simulink model "test03"  (R2022a)
settings: Solver=FixedStepAuto  StartTime=0.0  StopTime=0.05  FixedStep=1e-4  RelTol=1e-3
values from neighbouring files (symbols this model uses):
  A = 0.01    [parameter_load3.m]
  d = 2.6    [parameter_load3.m]
  I = 3055e-8    (= 3.055e-05)    [parameter_load3.m]
  jv = 1064400    [parameter_load3.m]
  kf = 6.67e7    (= 66700000)    [parameter_load3.m]
  kv = 400000    [parameter_load3.m]
  L = 210    [parameter_load3.m]
  m = 7800*(7684e-6)    (= 59.9352)    [parameter_load3.m]
  mv = 42400    [parameter_load3.m]
  position = 0.5*L    (= 105)    [parameter_load3.m]
  r = 840/1000/2    (= 0.42)    [parameter_load3.m]
  v = 100/3.6    (= 27.7777777778)    [parameter_load3.m]
(part 2 of 73)

== system test03/Subsystem3/Subsystem1/Subsystem17 ==
  index : Inport
  F : Inport  Port=2
  Add1 : Sum
  Constant2 : Constant  Value=2
  Constant6 : Constant
  Constant7 : Constant  Value=2
  Constant8 : Constant  Value=2
  Demux : Demux  Outputs=2
  Subsystem : Subsystem
  Subsystem1 : Subsystem
  Subsystem2 : Subsystem
  Subsystem3 : Subsystem
  Out1 : Outport
  wires:
  Subsystem3/1(Out1) -> Add1/2
  Subsystem2/1(Out1) -> Add1/1
  Subsystem1/8(Omiga_n) -> Subsystem3/8(Omiga_n)
  Subsystem1/7(Omiga_bn) -> Subsystem3/7(Omiga_bn)
  Subsystem1/6(static_n) -> Subsystem3/6(static_n)
  Subsystem1/5(Sn) -> Subsystem3/5(Sn)
  Subsystem1/4(theta_n3) -> Subsystem3/4(theta_n3)
  Subsystem1/3(theta_n2) -> Subsystem3/3(theta_n2)
  Subsystem1/2(theta_n1) -> Subsystem3/2(theta_n1)
  Subsystem1/1(An1) -> Subsystem3/1(An1)
  Subsystem/8(Omiga_n) -> Subsystem2/8(Omiga_n)
  Subsystem/7(Omiga_bn) -> Subsystem2/7(Omiga_bn)
  Subsystem/6(static_n) -> Subsystem2/6(static_n)
  Subsystem/5(Sn) -> Subsystem2/5(Sn)
  Subsystem/1(An1) -> Subsystem2/1(An1)
  Subsystem/4(theta_n3) -> Subsystem2/4(theta_n3)
  Subsystem/3(theta_n2) -> Subsystem2/3(theta_n2)
  Subsystem/2(theta_n1) -> Subsystem2/2(theta_n1)
  Demux/2 -> Subsystem1/4(F)
  Demux/1 -> Subsystem/4(F)
  F/1 -> Demux/1
  Constant7/1 -> Subsystem1/2(r)
  Constant8/1 -> Subsystem1/3(k)
  Constant6/1 -> Subsystem/3(k)
  Constant2/1 -> Subsystem/2(r)
  Add1/1 -> Out1/1
  index/1 -> Subsystem1/1(imod), Subsystem/1(imod)

== system test03/Subsystem3/Subsystem1/Subsystem18 ==
  index : Inport
  F : Inport  Port=2
  Add1 : Sum
  Constant2 : Constant  Value=2
  Constant6 : Constant
  Constant7 : Constant  Value=2
  Constant8 : Constant  Value=2
  Demux : Demux  Outputs=2
  Subsystem : Subsystem
  Subsystem1 : Subsystem
  Subsystem2 : Subsystem
  Subsystem3 : Subsystem
  Out1 : Outport
  wires:
  Subsystem3/1(Out1) -> Add1/2
  Subsystem2/1(Out1) -> Add1/1
  Subsystem1/8(Omiga_n) -> Subsystem3/8(Omiga_n)
  Subsystem1/7(Omiga_bn) -> Subsystem3/7(Omiga_bn)
  Subsystem1/6(static_n) -> Subsystem3/6(static_n)
  Subsystem1/5(Sn) -> Subsystem3/5(Sn)
  Subsystem1/4(theta_n3) -> Subsystem3/4(theta_n3)
  Subsystem1/3(theta_n2) -> Subsystem3/3(theta_n2)
  Subsystem1/2(theta_n1) -> Subsystem3/2(theta_n1)
  Subsystem1/1(An1) -> Subsystem3/1(An1)
  Subsystem/8(Omiga_n) -> Subsystem2/8(Omiga_n)
  Subsystem/7(Omiga_bn) -> Subsystem2/7(Omiga_bn)
  Subsystem/6(static_n) -> Subsystem2/6(static_n)
  Subsystem/5(Sn) -> Subsystem2/5(Sn)
  Subsystem/1(An1) -> Subsystem2/1(An1)
  Subsystem/4(theta_n3) -> Subsystem2/4(theta_n3)
  Subsystem/3(theta_n2) -> Subsystem2/3(theta_n2)
  Subsystem/2(theta_n1) -> Subsystem2/2(theta_n1)
  Demux/2 -> Subsystem1/4(F)
  Demux/1 -> Subsystem/4(F)
  F/1 -> Demux/1
  Constant7/1 -> Subsystem1/2(r)
  Constant8/1 -> Subsystem1/3(k)
  Constant6/1 -> Subsystem/3(k)
  Constant2/1 -> Subsystem/2(r)
  Add1/1 -> Out1/1
  index/1 -> Subsystem1/1(imod), Subsystem/1(imod)

== system test03/Subsystem3/Subsystem1/Subsystem19 ==
  index : Inport
  F : Inport  Port=2
  Add1 : Sum
  Constant2 : Constant  Value=2
  Constant6 : Constant
  Constant7 : Constant  Value=2
  Constant8 : Constant  Value=2
  Demux : Demux  Outputs=2
  Subsystem : Subsystem
  Subsystem1 : Subsystem
  Subsystem2 : Subsystem
  Subsystem3 : Subsystem
  Out1 : Outport
  wires:
  index/1 -> Subsystem/1(imod), Subsystem1/1(imod)
  Add1/1 -> Out1/1
  Constant2/1 -> Subsystem/2(r)
  Constant6/1 -> Subsystem/3(k)
  Constant8/1 -> Subsystem1/3(k)
  Constant7/1 -> Subsystem1/2(r)
  F/1 -> Demux/1
  Demux/1 -> Subsystem/4(F)
  Demux/2 -> Subsystem1/4(F)
  Subsystem/2(theta_n1) -> Subsystem2/2(theta_n1)
  Subsystem/3(theta_n2) -> Subsystem2/3(theta_n2)
  Subsystem/4(theta_n3) -> Subsystem2/4(theta_n3)
  Subsystem/1(An1) -> Subsystem2/1(An1)
  Subsystem/5(Sn) -> Subsystem2/5(Sn)
  Subsystem/6(static_n) -> Subsystem2/6(static_n)
  Subsystem/7(Omiga_bn) -> Subsystem2/7(Omiga_bn)
  Subsystem/8(Omiga_n) -> Subsystem2/8(Omiga_n)
  Subsystem1/1(An1) -> Subsystem3/1(An1)
  Subsystem1/2(theta_n1) -> Subsystem3/2(theta_n1)
  Subsystem1/3(theta_n2) -> Subsystem3/3(theta_n2)
  Subsystem1/4(theta_n3) -> Subsystem3/4(theta_n3)
  Subsystem1/5(Sn) -> Subsystem3/5(Sn)
  Subsystem1/6(static_n) -> Subsystem3/6(static_n)
  Subsystem1/7(Omiga_bn) -> Subsystem3/7(Omiga_bn)
  Subsystem1/8(Omiga_n) -> Subsystem3/8(Omiga_n)
  Subsystem2/1(Out1) -> Add1/1
  Subsystem3/1(Out1) -> Add1/2

== system test03/Subsystem3/Subsystem1/Subsystem2 ==
  index : Inport
  F : Inport  Port=2
  Add1 : Sum
  Constant2 : Constant  Value=2
  Constant6 : Constant
  Constant7 : Constant  Value=2
  Constant8 : Constant  Value=2
  Demux : Demux  Outputs=2
  Subsystem : Subsystem
  Subsystem1 : Subsystem
  Subsystem2 : Subsystem
  Subsystem3 : Subsystem
  Out1 : Outport
  wires:
  index/1 -> Subsystem/1(imod), Subsystem1/1(imod)
  Add1/1 -> Out1/1
  Constant2/1 -> Subsystem/2(r)
  Constant6/1 -> Subsystem/3(k)
  Constant8/1 -> Subsystem1/3(k)
  Constant7/1 -> Subsystem1/2(r)
  F/1 -> Demux/1
  Demux/1 -> Subsystem/4(F)
  Demux/2 -> Subsystem1/4(F)
  Subsystem/2(theta_n1) -> Subsystem2/2(theta_n1)
  Subsystem/3(theta_n2) -> Subsystem2/3(theta_n2)
  Subsystem/4(theta_n3) -> Subsystem2/4(theta_n3)
  Subsystem/1(An1) -> Subsystem2/1(An1)
  Subsystem/5(Sn) -> Subsystem2/5(Sn)
  Subsystem/6(static_n) -> Subsystem2/6(static_n)
  Subsystem/7(Omiga_bn) -> Subsystem2/7(Omiga_bn)
  Subsystem/8(Omiga_n) -> Subsystem2/8(Omiga_n)
  Subsystem1/1(An1) -> Subsystem3/1(An1)
  Subsystem1/2(theta_n1) -> Subsystem3/2(theta_n1)
  Subsystem1/3(theta_n2) -> Subsystem3/3(theta_n2)
  Subsystem1/4(theta_n3) -> Subsystem3/4(theta_n3)
  Subsystem1/5(Sn) -> Subsystem3/5(Sn)
  Subsystem1/6(static_n) -> Subsystem3/6(static_n)
  Subsystem1/7(Omiga_bn) -> Subsystem3/7(Omiga_bn)
  Subsystem1/8(Omiga_n) -> Subsystem3/8(Omiga_n)
  Subsystem2/1(Out1) -> Add1/1
  Subsystem3/1(Out1) -> Add1/2

== system test03/Subsystem3/Subsystem1/Subsystem20 ==
  index : Inport
  F : Inport  Port=2
  Add1 : Sum
  Constant2 : Constant  Value=2
  Constant6 : Constant
  Constant7 : Constant  Value=2
  Constant8 : Constant  Value=2
  Demux : Demux  Outputs=2
  Subsystem : Subsystem
  Subsystem1 : Subsystem
  Subsystem2 : Subsystem
  Subsystem3 : Subsystem
  Out1 : Outport
  wires:
  Subsystem3/1(Out1) -> Add1/2
  Subsystem2/1(Out1) -> Add1/1
  Subsystem1/8(Omiga_n) -> Subsystem3/8(Omiga_n)
  Subsystem1/7(Omiga_bn) -> Subsystem3/7(Omiga_bn)
  Subsystem1/6(static_n) -> Subsystem3/6(static_n)
  Subsystem1/5(Sn) -> Subsystem3/5(Sn)
  Subsystem1/4(theta_n3) -> Subsystem3/4(theta_n3)
  Subsystem1/3(theta_n2) -> Subsystem3/3(theta_n2)
  Subsystem1/2(theta_n1) -> Subsystem3/2(theta_n1)
  Subsystem1/1(An1) -> Subsystem3/1(An1)
  Subsystem/8(Omiga_n) -> Subsystem2/8(Omiga_n)
  Subsystem/7(Omiga_bn) -> Subsystem2/7(Omiga_bn)
  Subsystem/6(static_n) -> Subsystem2/6(static_n)
  Subsystem/5(Sn) -> Subsystem2/5(Sn)
  Subsystem/1(An1) -> Subsystem2/1(An1)
  Subsystem/4(theta_n3) -> Subsystem2/4(theta_n3)
  Subsystem/3(theta_n2) -> Subsystem2/3(theta_n2)
  Subsystem/2(theta_n1) -> Subsystem2/2(theta_n1)
  Demux/2 -> Subsystem1/4(F)
  Demux/1 -> Subsystem/4(F)
  F/1 -> Demux/1
  Constant7/1 -> Subsystem1/2(r)
  Constant8/1 -> Subsystem1/3(k)
  Constant6/1 -> Subsystem/3(k)
  Constant2/1 -> Subsystem/2(r)
  Add1/1 -> Out1/1
  index/1 -> Subsystem1/1(imod), Subsystem/1(imod)

== system test03/Subsystem3/Subsystem1/Subsystem3 ==
  index : Inport
  F : Inport  Port=2
  Add1 : Sum
  Constant2 : Constant  Value=2
  Constant6 : Constant
  Constant7 : Constant  Value=2
  Constant8 : Constant  Value=2
  Demux : Demux  Outputs=2
  Subsystem : Subsystem
  Subsystem1 : Subsystem
  Subsystem2 : Subsystem
  Subsystem3 : Subsystem
  Out1 : Outport
  wires:
  Subsystem3/1(Out1) -> Add1/2
  Subsystem2/1(Out1) -> Add1/1
  Subsystem1/8(Omiga_n) -> Subsystem3/8(Omiga_n)
  Subsystem1/7(Omiga_bn) -> Subsystem3/7(Omiga_bn)
  Subsystem1/6(static_n) -> Subsystem3/6(static_n)
  Subsystem1/5(Sn) -> Subsystem3/5(Sn)
  Subsystem1/4(theta_n3) -> Subsystem3/4(theta_n3)
  Subsystem1/3(theta_n2) -> Subsystem3/3(theta_n2)
  Subsystem1/2(theta_n1) -> Subsystem3/2(theta_n1)
  Subsystem1/1(An1) -> Subsystem3/1(An1)
  Subsystem/8(Omiga_n) -> Subsystem2/8(Omiga_n)
  Subsystem/7(Omiga_bn) -> Subsystem2/7(Omiga_bn)
  Subsystem/6(static_n) -> Subsystem2/6(static_n)
  Subsystem/5(Sn) -> Subsystem2/5(Sn)
  Subsystem/1(An1) -> Subsystem2/1(An1)
  Subsystem/4(theta_n3) -> Subsystem2/4(theta_n3)
  Subsystem/3(theta_n2) -> Subsystem2/3(theta_n2)
  Subsystem/2(theta_n1) -> Subsystem2/2(theta_n1)
  Demux/2 -> Subsystem1/4(F)
  Demux/1 -> Subsystem/4(F)
  F/1 -> Demux/1
  Constant7/1 -> Subsystem1/2(r)
  Constant8/1 -> Subsystem1/3(k)
  Constant6/1 -> Subsystem/3(k)
  Constant2/1 -> Subsystem/2(r)
  Add1/1 -> Out1/1
  index/1 -> Subsystem1/1(imod), Subsystem/1(imod)

== system test03/Subsystem3/Subsystem1/Subsystem4 ==
  index : Inport
  F : Inport  Port=2
  Add1 : Sum
  Constant2 : Constant  Value=2
  Constant6 : Constant
  Constant7 : Constant  Value=2
  Constant8 : Constant  Value=2
  Demux : Demux  Outputs=2
  Subsystem : Subsystem
  Subsystem1 : Subsystem
  Subsystem2 : Subsystem
  Subsystem3 : Subsystem
  Out1 : Outport
  wires:
  index/1 -> Subsystem/1(imod), Subsystem1/1(imod)
  Add1/1 -> Out1/1
  Constant2/1 -> Subsystem/2(r)
  Constant6/1 -> Subsystem/3(k)
  Constant8/1 -> Subsystem1/3(k)
  Constant7/1 -> Subsystem1/2(r)
  F/1 -> Demux/1
  Demux/1 -> Subsystem/4(F)
  Demux/2 -> Subsystem1/4(F)
  Subsystem/2(theta_n1) -> Subsystem2/2(theta_n1)
  Subsystem/3(theta_n2) -> Subsystem2/3(theta_n2)
  Subsystem/4(theta_n3) -> Subsystem2/4(theta_n3)
  Subsystem/1(An1) -> Subsystem2/1(An1)
  Subsystem/5(Sn) -> Subsystem2/5(Sn)
  Subsystem/6(static_n) -> Subsystem2/6(static_n)
  Subsystem/7(Omiga_bn) -> Subsystem2/7(Omiga_bn)
  Subsystem/8(Omiga_n) -> Subsystem2/8(Omiga_n)
  Subsystem1/1(An1) -> Subsystem3/1(An1)
  Subsystem1/2(theta_n1) -> Subsystem3/2(theta_n1)
  Subsystem1/3(theta_n2) -> Subsystem3/3(theta_n2)
  Subsystem1/4(theta_n3) -> Subsystem3/4(theta_n3)
  Subsystem1/5(Sn) -> Subsystem3/5(Sn)
  Subsystem1/6(static_n) -> Subsystem3/6(static_n)
  Subsystem1/7(Omiga_bn) -> Subsystem3/7(Omiga_bn)
  Subsystem1/8(Omiga_n) -> Subsystem3/8(Omiga_n)
  Subsystem2/1(Out1) -> Add1/1
  Subsystem3/1(Out1) -> Add1/2

== system test03/Subsystem3/Subsystem1/Subsystem5 ==
  index : Inport
  F : Inport  Port=2
  Add1 : Sum
  Constant2 : Constant  Value=2
  Constant6 : Constant
  Constant7 : Constant  Value=2
  Constant8 : Constant  Value=2
  Demux : Demux  Outputs=2
  Subsystem : Subsystem
  Subsystem1 : Subsystem
  Subsystem2 : Subsystem
  Subsystem3 : Subsystem
  Out1 : Outport
  wires:
  Subsystem3/1(Out1) -> Add1/2
  Subsystem2/1(Out1) -> Add1/1
  Subsystem1/8(Omiga_n) -> Subsystem3/8(Omiga_n)
  Subsystem1/7(Omiga_bn) -> Subsystem3/7(Omiga_bn)
  Subsystem1/6(static_n) -> Subsystem3/6(static_n)
  Subsystem1/5(Sn) -> Subsystem3/5(Sn)
  Subsystem1/4(theta_n3) -> Subsystem3/4(theta_n3)
  Subsystem1/3(theta_n2) -> Subsystem3/3(theta_n2)
  Subsystem1/2(theta_n1) -> Subsystem3/2(theta_n1)
  Subsystem1/1(An1) -> Subsystem3/1(An1)
  Subsystem/8(Omiga_n) -> Subsystem2/8(Omiga_n)
  Subsystem/7(Omiga_bn) -> Subsystem2/7(Omiga_bn)
  Subsystem/6(static_n) -> Subsystem2/6(static_n)
  Subsystem/5(Sn) -> Subsystem2/5(Sn)
  Subsystem/1(An1) -> Subsystem2/1(An1)
  Subsystem/4(theta_n3) -> Subsystem2/4(theta_n3)
  Subsystem/3(theta_n2) -> Subsystem2/3(theta_n2)
  Subsystem/2(theta_n1) -> Subsystem2/2(theta_n1)
  Demux/2 -> Subsystem1/4(F)
  Demux/1 -> Subsystem/4(F)
  F/1 -> Demux/1
  Constant7/1 -> Subsystem1/2(r)
  Constant8/1 -> Subsystem1/3(k)
  Constant6/1 -> Subsystem/3(k)
  Constant2/1 -> Subsystem/2(r)
  Add1/1 -> Out1/1
  index/1 -> Subsystem1/1(imod), Subsystem/1(imod)

== system test03/Subsystem3/Subsystem1/Subsystem6 ==
  index : Inport
  F : Inport  Port=2
  Add1 : Sum
  Constant2 : Constant  Value=2
  Constant6 : Constant
  Constant7 : Constant  Value=2
  Constant8 : Constant  Value=2
  Demux : Demux  Outputs=2
  Subsystem : Subsystem
  Subsystem1 : Subsystem
  Subsystem2 : Subsystem
  Subsystem3 : Subsystem
  Out1 : Outport
  wires:
  index/1 -> Subsystem/1(imod), Subsystem1/1(imod)
  Add1/1 -> Out1/1
  Constant2/1 -> Subsystem/2(r)
  Constant6/1 -> Subsystem/3(k)
  Constant8/1 -> Subsystem1/3(k)
  Constant7/1 -> Subsystem1/2(r)
  F/1 -> Demux/1
  Demux/1 -> Subsystem/4(F)
  Demux/2 -> Subsystem1/4(F)
  Subsystem/2(theta_n1) -> Subsystem2/2(theta_n1)
  Subsystem/3(theta_n2) -> Subsystem2/3(theta_n2)
  Subsystem/4(theta_n3) -> Subsystem2/4(theta_n3)
  Subsystem/1(An1) -> Subsystem2/1(An1)
  Subsystem/5(Sn) -> Subsystem2/5(Sn)
  Subsystem/6(static_n) -> Subsystem2/6(static_n)
  Subsystem/7(Omiga_bn) -> Subsystem2/7(Omiga_bn)
  Subsystem/8(Omiga_n) -> Subsystem2/8(Omiga_n)
  Subsystem1/1(An1) -> Subsystem3/1(An1)
  Subsystem1/2(theta_n1) -> Subsystem3/2(theta_n1)
  Subsystem1/3(theta_n2) -> Subsystem3/3(theta_n2)
  Subsystem1/4(theta_n3) -> Subsystem3/4(theta_n3)
  Subsystem1/5(Sn) -> Subsystem3/5(Sn)
  Subsystem1/6(static_n) -> Subsystem3/6(static_n)
  Subsystem1/7(Omiga_bn) -> Subsystem3/7(Omiga_bn)
  Subsystem1/8(Omiga_n) -> Subsystem3/8(Omiga_n)
  Subsystem2/1(Out1) -> Add1/1
  Subsystem3/1(Out1) -> Add1/2

== system test03/Subsystem3/Subsystem1/Subsystem7 ==
  index : Inport
  F : Inport  Port=2
  Add1 : Sum
  Constant2 : Constant  Value=2
  Constant6 : Constant
  Constant7 : Constant  Value=2
  Constant8 : Constant  Value=2
  Demux : Demux  Outputs=2
  Subsystem : Subsystem
  Subsystem1 : Subsystem
  Subsystem2 : Subsystem
  Subsystem3 : Subsystem
  Out1 : Outport
  wires:
  index/1 -> Subsystem/1(imod), Subsystem1/1(imod)
  Add1/1 -> Out1/1
  Constant2/1 -> Subsystem/2(r)
  Constant6/1 -> Subsystem/3(k)
  Constant8/1 -> Subsystem1/3(k)
  Constant7/1 -> Subsystem1/2(r)
  F/1 -> Demux/1
  Demux/1 -> Subsystem/4(F)
  Demux/2 -> Subsystem1/4(F)
  Subsystem/2(theta_n1) -> Subsystem2/2(theta_n1)
  Subsystem/3(theta_n2) -> Subsystem2/3(theta_n2)
  Subsystem/4(theta_n3) -> Subsystem2/4(theta_n3)
  Subsystem/1(An1) -> Subsystem2/1(An1)
  Subsystem/5(Sn) -> Subsystem2/5(Sn)
  Subsystem/6(static_n) -> Subsystem2/6(static_n)
  Subsystem/7(Omiga_bn) -> Subsystem2/7(Omiga_bn)
  Subsystem/8(Omiga_n) -> Subsystem2/8(Omiga_n)
  Subsystem1/1(An1) -> Subsystem3/1(An1)
  Subsystem1/2(theta_n1) -> Subsystem3/2(theta_n1)
  Subsystem1/3(theta_n2) -> Subsystem3/3(theta_n2)
  Subsystem1/4(theta_n3) -> Subsystem3/4(theta_n3)
  Subsystem1/5(Sn) -> Subsystem3/5(Sn)
  Subsystem1/6(static_n) -> Subsystem3/6(static_n)
  Subsystem1/7(Omiga_bn) -> Subsystem3/7(Omiga_bn)
  Subsystem1/8(Omiga_n) -> Subsystem3/8(Omiga_n)
  Subsystem2/1(Out1) -> Add1/1
  Subsystem3/1(Out1) -> Add1/2

== system test03/Subsystem3/Subsystem1/Subsystem8 ==
  index : Inport
  F : Inport  Port=2
  Add1 : Sum
  Constant2 : Constant  Value=2
  Constant6 : Constant
  Constant7 : Constant  Value=2
  Constant8 : Constant  Value=2
  Demux : Demux  Outputs=2
  Subsystem : Subsystem
  Subsystem1 : Subsystem
  Subsystem2 : Subsystem
  Subsystem3 : Subsystem
  Out1 : Outport
  wires:
  Subsystem3/1(Out1) -> Add1/2
  Subsystem2/1(Out1) -> Add1/1
  Subsystem1/8(Omiga_n) -> Subsystem3/8(Omiga_n)
  Subsystem1/7(Omiga_bn) -> Subsystem3/7(Omiga_bn)
  Subsystem1/6(static_n) -> Subsystem3/6(static_n)
  Subsystem1/5(Sn) -> Subsystem3/5(Sn)
  Subsystem1/4(theta_n3) -> Subsystem3/4(theta_n3)
  Subsystem1/3(theta_n2) -> Subsystem3/3(theta_n2)
  Subsystem1/2(theta_n1) -> Subsystem3/2(theta_n1)
  Subsystem1/1(An1) -> Subsystem3/1(An1)
  Subsystem/8(Omiga_n) -> Subsystem2/8(Omiga_n)
  Subsystem/7(Omiga_bn) -> Subsystem2/7(Omiga_bn)
  Subsystem/6(static_n) -> Subsystem2/6(static_n)
  Subsystem/5(Sn) -> Subsystem2/5(Sn)
  Subsystem/1(An1) -> Subsystem2/1(An1)
  Subsystem/4(theta_n3) -> Subsystem2/4(theta_n3)
  Subsystem/3(theta_n2) -> Subsystem2/3(theta_n2)
  Subsystem/2(theta_n1) -> Subsystem2/2(theta_n1)
  Demux/2 -> Subsystem1/4(F)
  Demux/1 -> Subsystem/4(F)
  F/1 -> Demux/1
  Constant7/1 -> Subsystem1/2(r)
  Constant8/1 -> Subsystem1/3(k)
  Constant6/1 -> Subsystem/3(k)
  Constant2/1 -> Subsystem/2(r)
  Add1/1 -> Out1/1
  index/1 -> Subsystem1/1(imod), Subsystem/1(imod)

== system test03/Subsystem3/Subsystem1/Subsystem9 ==
  index : Inport
  F : Inport  Port=2
  Add1 : Sum
  Constant2 : Constant  Value=2
  Constant6 : Constant
  Constant7 : Constant  Value=2
  Constant8 : Constant  Value=2
  Demux : Demux  Outputs=2
  Subsystem : Subsystem
  Subsystem1 : Subsystem
  Subsystem2 : Subsystem
  Subsystem3 : Subsystem
  Out1 : Outport
  wires:
  index/1 -> Subsystem/1(imod), Subsystem1/1(imod)
  Add1/1 -> Out1/1
  Constant2/1 -> Subsystem/2(r)
  Constant6/1 -> Subsystem/3(k)
  Constant8/1 -> Subsystem1/3(k)
  Constant7/1 -> Subsystem1/2(r)
  F/1 -> Demux/1
  Demux/1 -> Subsystem/4(F)
  Demux/2 -> Subsystem1/4(F)
  Subsystem/2(theta_n1) -> Subsystem2/2(theta_n1)
  Subsystem/3(theta_n2) -> Subsystem2/3(theta_n2)
  Subsystem/4(theta_n3) -> Subsystem2/4(theta_n3)
  Subsystem/1(An1) -> Subsystem2/1(An1)
  Subsystem/5(Sn) -> Subsystem2/5(Sn)
  Subsystem/6(static_n) -> Subsystem2/6(static_n)
  Subsystem/7(Omiga_bn) -> Subsystem2/7(Omiga_bn)
  Subsystem/8(Omiga_n) -> Subsystem2/8(Omiga_n)
  Subsystem1/1(An1) -> Subsystem3/1(An1)
  Subsystem1/2(theta_n1) -> Subsystem3/2(theta_n1)
  Subsystem1/3(theta_n2) -> Subsystem3/3(theta_n2)
  Subsystem1/4(theta_n3) -> Subsystem3/4(theta_n3)
  Subsystem1/5(Sn) -> Subsystem3/5(Sn)
  Subsystem1/6(static_n) -> Subsystem3/6(static_n)
  Subsystem1/7(Omiga_bn) -> Subsystem3/7(Omiga_bn)
  Subsystem1/8(Omiga_n) -> Subsystem3/8(Omiga_n)
  Subsystem2/1(Out1) -> Add1/1
  Subsystem3/1(Out1) -> Add1/2

== system test03/Subsystem3/Subsystem3/Subsystem ==
  In2 : Inport
  In1 : Inport  Port=2
  Add : Sum  Inputs=++++++++++++++++++++
  Add Constant{,1..16,19..20} (19 alike) : Bias  Bias=1  SaturateOnIntegerOverflow=off
  Subsystem1 : Subsystem
  Subsystem10 : Subsystem
  Subsystem11 : Subsystem
  Subsystem12 : Subsystem
  Subsystem13 : Subsystem
  Subsystem14 : Subsystem
  Subsystem15 : Subsystem
  Subsystem16 : Subsystem
  Subsystem17 : Subsystem
  Subsystem18 : Subsystem
  Subsystem19 : Subsystem
  Subsystem2 : Subsystem
  Subsystem20 : Subsystem
  Subsystem3 : Subsystem
  Subsystem4 : Subsystem
  Subsystem5 : Subsystem
  Subsystem6 : Subsystem
  Subsystem7 : Subsystem
  Subsystem8 : Subsystem
  Subsystem9 : Subsystem
  Out1 : Outport
  wires:
  In2/1 -> [Ff]
  Subsystem12/1(Out1) -> Add/20
  Subsystem18/1(Out1) -> Add/17
  [Ff] -> Subsystem3/2(F)
  [Ff] -> Subsystem17/2(F)
  Subsystem9/1(Out1) -> Add/9
  [Ff] -> Subsystem9/2(F)
  Add Constant7/1 -> Add Constant8/1, Subsystem9/1(index)
  [Ff] -> Subsystem5/2(F)
  Subsystem7/1(Out1) -> Add/7
  Add Constant10/1 -> Subsystem13/1(index), Add Constant11/1
  [Ff] -> Subsystem15/2(F)
  Add Constant8/1 -> Subsystem10/1(index), Add Constant9/1
  Subsystem10/1(Out1) -> Add/10
  Add Constant1/1 -> Subsystem3/1(index), Add Constant2/1
  Subsystem17/1(Out1) -> Add/16
  [Ff] -> Subsystem12/2(F)
  Add/1 -> Out1/1
  Add Constant11/1 -> Subsystem14/1(index), Add Constant14/1
  [Ff] -> Subsystem16/2(F)
  In1/1 -> Add Constant/1, Subsystem1/1(index)
  Subsystem14/1(Out1) -> Add/13
  Add Constant12/1 -> Subsystem18/1(index), Add Constant13/1
  Subsystem6/1(Out1) -> Add/6
  Add Constant5/1 -> Subsystem7/1(index), Add Constant6/1
  [Ff] -> Subsystem1/2(F)
  Add Constant4/1 -> Subsystem6/1(index), Add Constant5/1
  [Ff] -> Subsystem18/2(F)
  [Ff] -> Subsystem2/2(F)
  Add Constant20/1 -> Subsystem12/1(index)
  [Ff] -> Subsystem6/2(F)
  Add Constant15/1 -> Subsystem16/1(index), Add Constant16/1
  [Ff] -> Subsystem19/2(F)
  Subsystem16/1(Out1) -> Add/15
  Subsystem20/1(Out1) -> Add/19
  [Ff] -> Subsystem10/2(F)
  [Ff] -> Subsystem13/2(F)
  Add Constant14/1 -> Subsystem15/1(index), Add Constant15/1
  [Ff] -> Subsystem7/2(F)
  Add Constant6/1 -> Subsystem8/1(index), Add Constant7/1
  Add Constant/1 -> Subsystem2/1(index), Add Constant1/1
  Subsystem2/1(Out1) -> Add/2
  [Ff] -> Subsystem11/2(F)
  Subsystem11/1(Out1) -> Add/11
  [Ff] -> Subsystem4/2(F)
  Subsystem15/1(Out1) -> Add/14
  Add Constant2/1 -> Subsystem4/1(index), Add Constant3/1
  Add Constant16/1 -> Subsystem17/1(index), Add Constant12/1
  Subsystem4/1(Out1) -> Add/4
  [Ff] -> Subsystem8/2(F)
  Subsystem3/1(Out1) -> Add/3
  Subsystem5/1(Out1) -> Add/5
  Subsystem8/1(Out1) -> Add/8
  [Ff] -> Subsystem14/2(F)
  Add Constant19/1 -> Add Constant20/1, Subsystem20/1(index)
  Add Constant9/1 -> Subsystem11/1(index), Add Constant10/1
  Add Constant3/1 -> Add Constant4/1, Subsystem5/1(index)
  Add Constant13/1 -> Subsystem19/1(index), Add Constant19/1
  Subsystem19/1(Out1) -> Add/18
  Subsystem13/1(Out1) -> Add/12
  [Ff] -> Subsystem20/2(F)
  Subsystem1/1(Out1) -> Add/1

== system test03/Subsystem3/Subsystem3/Subsystem1 ==
  In2 : Inport
  In1 : Inport  Port=2
  Add : Sum  Inputs=++++++++++++++++++++
  Add Constant{,1..16,19..20} (19 alike) : Bias  Bias=1  SaturateOnIntegerOverflow=off
  Subsystem1 : Subsystem
  Subsystem10 : Subsystem
  Subsystem11 : Subsystem
  Subsystem12 : Subsystem
  Subsystem13 : Subsystem
  Subsystem14 : Subsystem
  Subsystem15 : Subsystem
  Subsystem16 : Subsystem
  Subsystem17 : Subsystem
  Subsystem18 : Subsystem
  Subsystem19 : Subsystem
  Subsystem2 : Subsystem
  Subsystem20 : Subsystem
  Subsystem3 : Subsystem
  Subsystem4 : Subsystem
  Subsystem5 : Subsystem
  Subsystem6 : Subsystem
  Subsystem7 : Subsystem
  Subsystem8 : Subsystem
  Subsystem9 : Subsystem
  Out1 : Outport
  wires:
  In2/1 -> [Ff]
  Subsystem1/1(Out1) -> Add/1
  [Ff] -> Subsystem20/2(F)
  Subsystem13/1(Out1) -> Add/12
  Subsystem19/1(Out1) -> Add/18
  Add Constant13/1 -> Add Constant19/1, Subsystem19/1(index)
  Add Constant3/1 -> Subsystem5/1(index), Add Constant4/1
  Add Constant9/1 -> Add Constant10/1, Subsystem11/1(index)
  Add Constant19/1 -> Subsystem20/1(index), Add Constant20/1
  [Ff] -> Subsystem14/2(F)
  Subsystem8/1(Out1) -> Add/8
  Subsystem5/1(Out1) -> Add/5
  Subsystem3/1(Out1) -> Add/3
  [Ff] -> Subsystem8/2(F)
  Subsystem4/1(Out1) -> Add/4
  Add Constant16/1 -> Add Constant12/1, Subsystem17/1(index)
  Add Constant2/1 -> Add Constant3/1, Subsystem4/1(index)
  Subsystem15/1(Out1) -> Add/14
  [Ff] -> Subsystem4/2(F)
  Subsystem11/1(Out1) -> Add/11
  [Ff] -> Subsystem11/2(F)
  Subsystem2/1(Out1) -> Add/2
  Add Constant/1 -> Add Constant1/1, Subsystem2/1(index)
  Add Constant6/1 -> Add Constant7/1, Subsystem8/1(index)
  [Ff] -> Subsystem7/2(F)
  Add Constant14/1 -> Add Constant15/1, Subsystem15/1(index)
  [Ff] -> Subsystem13/2(F)
  [Ff] -> Subsystem10/2(F)
  Subsystem20/1(Out1) -> Add/19
  Subsystem16/1(Out1) -> Add/15
  [Ff] -> Subsystem19/2(F)
  Add Constant15/1 -> Add Constant16/1, Subsystem16/1(index)
  [Ff] -> Subsystem6/2(F)
  Add Constant20/1 -> Subsystem12/1(index)
  [Ff] -> Subsystem2/2(F)
  [Ff] -> Subsystem18/2(F)
  Add Constant4/1 -> Add Constant5/1, Subsystem6/1(index)
  [Ff] -> Subsystem1/2(F)
  Add Constant5/1 -> Add Constant6/1, Subsystem7/1(index)
  Subsystem6/1(Out1) -> Add/6
  Add Constant12/1 -> Add Constant13/1, Subsystem18/1(index)
  Subsystem14/1(Out1) -> Add/13
  In1/1 -> Subsystem1/1(index), Add Constant/1
  [Ff] -> Subsystem16/2(F)
  Add Constant11/1 -> Add Constant14/1, Subsystem14/1(index)
  Add/1 -> Out1/1
  [Ff] -> Subsystem12/2(F)
  Subsystem17/1(Out1) -> Add/16
  Add Constant1/1 -> Add Constant2/1, Subsystem3/1(index)
  Subsystem10/1(Out1) -> Add/10
  Add Constant8/1 -> Add Constant9/1, Subsystem10/1(index)
  [Ff] -> Subsystem15/2(F)
  Add Constant10/1 -> Add Constant11/1, Subsystem13/1(index)
  Subsystem7/1(Out1) -> Add/7
  [Ff] -> Subsystem5/2(F)
  Add Constant7/1 -> Subsystem9/1(index), Add Constant8/1
  [Ff] -> Subsystem9/2(F)
  Subsystem9/1(Out1) -> Add/9
  [Ff] -> Subsystem17/2(F)
  [Ff] -> Subsystem3/2(F)
  Subsystem18/1(Out1) -> Add/17
  Subsystem12/1(Out1) -> Add/20

== system test03/Subsystem3/Subsystem3/Subsystem2 ==
  In2 : Inport
  In1 : Inport  Port=2
  Add : Sum  Inputs=++++++++++++++++++++
  Add Constant{,1..16,19..20} (19 alike) : Bias  Bias=1  SaturateOnIntegerOverflow=off
  Subsystem1 : Subsystem
  Subsystem10 : Subsystem
  Subsystem11 : Subsystem
  Subsystem12 : Subsystem
  Subsystem13 : Subsystem
  Subsystem14 : Subsystem
  Subsystem15 : Subsystem
  Subsystem16 : Subsystem
  Subsystem17 : Subsystem
  Subsystem18 : Subsystem
  Subsystem19 : Subsystem
  Subsystem2 : Subsystem
  Subsystem20 : Subsystem
  Subsystem3 : Subsystem
  Subsystem4 : Subsystem
  Subsystem5 : Subsystem
  Subsystem6 : Subsystem
  Subsystem7 : Subsystem
  Subsystem8 : Subsystem
  Subsystem9 : Subsystem
  Out1 : Outport
  wires:
  In2/1 -> [Ff]
  Subsystem12/1(Out1) -> Add/20
  Subsystem18/1(Out1) -> Add/17
  [Ff] -> Subsystem3/2(F)
  [Ff] -> Subsystem17/2(F)
  Subsystem9/1(Out1) -> Add/9
  [Ff] -> Subsystem9/2(F)
  Add Constant7/1 -> Add Constant8/1, Subsystem9/1(index)
  [Ff] -> Subsystem5/2(F)
  Subsystem7/1(Out1) -> Add/7
  Add Constant10/1 -> Subsystem13/1(index), Add Constant11/1
  [Ff] -> Subsystem15/2(F)
  Add Constant8/1 -> Subsystem10/1(index), Add Constant9/1
  Subsystem10/1(Out1) -> Add/10
  Add Constant1/1 -> Subsystem3/1(index), Add Constant2/1
  Subsystem17/1(Out1) -> Add/16
  [Ff] -> Subsystem12/2(F)
  Add/1 -> Out1/1
  Add Constant11/1 -> Subsystem14/1(index), Add Constant14/1
  [Ff] -> Subsystem16/2(F)
  In1/1 -> Add Constant/1, Subsystem1/1(index)
  Subsystem14/1(Out1) -> Add/13
  Add Constant12/1 -> Subsystem18/1(index), Add Constant13/1
  Subsystem6/1(Out1) -> Add/6
  Add Constant5/1 -> Subsystem7/1(index), Add Constant6/1
  [Ff] -> Subsystem1/2(F)
  Add Constant4/1 -> Subsystem6/1(index), Add Constant5/1
  [Ff] -> Subsystem18/2(F)
  [Ff] -> Subsystem2/2(F)
  Add Constant20/1 -> Subsystem12/1(index)
  [Ff] -> Subsystem6/2(F)
  Add Constant15/1 -> Subsystem16/1(index), Add Constant16/1
  [Ff] -> Subsystem19/2(F)
  Subsystem16/1(Out1) -> Add/15
  Subsystem20/1(Out1) -> Add/19
  [Ff] -> Subsystem10/2(F)
  [Ff] -> Subsystem13/2(F)
  Add Constant14/1 -> Subsystem15/1(index), Add Constant15/1
  [Ff] -> Subsystem7/2(F)
  Add Constant6/1 -> Subsystem8/1(index), Add Constant7/1
  Add Constant/1 -> Subsystem2/1(index), Add Constant1/1
  Subsystem2/1(Out1) -> Add/2
  [Ff] -> Subsystem11/2(F)
  Subsystem11/1(Out1) -> Add/11
  [Ff] -> Subsystem4/2(F)
  Subsystem15/1(Out1) -> Add/14
  Add Constant2/1 -> Subsystem4/1(index), Add Constant3/1
  Add Constant16/1 -> Subsystem17/1(index), Add Constant12/1
  Subsystem4/1(Out1) -> Add/4
  [Ff] -> Subsystem8/2(F)
  Subsystem3/1(Out1) -> Add/3
  Subsystem5/1(Out1) -> Add/5
  Subsystem8/1(Out1) -> Add/8
  [Ff] -> Subsystem14/2(F)
  Add Constant19/1 -> Add Constant20/1, Subsystem20/1(index)
  Add Constant9/1 -> Subsystem11/1(index), Add Constant10/1
  Add Constant3/1 -> Add Constant4/1, Subsystem5/1(index)
  Add Constant13/1 -> Subsystem19/1(index), Add Constant19/1
  Subsystem19/1(Out1) -> Add/18
  Subsystem13/1(Out1) -> Add/12
  [Ff] -> Subsystem20/2(F)
  Subsystem1/1(Out1) -> Add/1

== system test03/Subsystem3/Subsystem3/Subsystem3 ==
  In2 : Inport
  In1 : Inport  Port=2
  Add : Sum  Inputs=++++++++++++++++++++
  Add Constant{,1..16,19..20} (19 alike) : Bias  Bias=1  SaturateOnIntegerOverflow=off
  Subsystem1 : Subsystem
  Subsystem10 : Subsystem
  Subsystem11 : Subsystem
  Subsystem12 : Subsystem
  Subsystem13 : Subsystem
  Subsystem14 : Subsystem
  Subsystem15 : Subsystem
  Subsystem16 : Subsystem
  Subsystem17 : Subsystem
  Subsystem18 : Subsystem
  Subsystem19 : Subsystem
  Subsystem2 : Subsystem
  Subsystem20 : Subsystem
  Subsystem3 : Subsystem
  Subsystem4 : Subsystem
  Subsystem5 : Subsystem
  Subsystem6 : Subsystem
  Subsystem7 : Subsystem
  Subsystem8 : Subsystem
  Subsystem9 : Subsystem
  Out1 : Outport
  wires:
  Subsystem1/1(Out1) -> Add/1
  [Ff] -> Subsystem20/2(F)
  Subsystem13/1(Out1) -> Add/12
  Subsystem19/1(Out1) -> Add/18
  Add Constant13/1 -> Add Constant19/1, Subsystem19/1(index)
  Add Constant3/1 -> Subsystem5/1(index), Add Constant4/1
  Add Constant9/1 -> Add Constant10/1, Subsystem11/1(index)
  Add Constant19/1 -> Subsystem20/1(index), Add Constant20/1
  [Ff] -> Subsystem14/2(F)
  Subsystem8/1(Out1) -> Add/8
  Subsystem5/1(Out1) -> Add/5
  Subsystem3/1(Out1) -> Add/3
  [Ff] -> Subsystem8/2(F)
  Subsystem4/1(Out1) -> Add/4
  Add Constant16/1 -> Add Constant12/1, Subsystem17/1(index)
  Add Constant2/1 -> Add Constant3/1, Subsystem4/1(index)
  Subsystem15/1(Out1) -> Add/14
  [Ff] -> Subsystem4/2(F)
  Subsystem11/1(Out1) -> Add/11
  [Ff] -> Subsystem11/2(F)
  Subsystem2/1(Out1) -> Add/2
  Add Constant/1 -> Add Constant1/1, Subsystem2/1(index)
  Add Constant6/1 -> Add Constant7/1, Subsystem8/1(index)
  [Ff] -> Subsystem7/2(F)
  Add Constant14/1 -> Add Constant15/1, Subsystem15/1(index)
  [Ff] -> Subsystem13/2(F)
  [Ff] -> Subsystem10/2(F)
  Subsystem20/1(Out1) -> Add/19
  Subsystem16/1(Out1) -> Add/15
  [Ff] -> Subsystem19/2(F)
  Add Constant15/1 -> Add Constant16/1, Subsystem16/1(index)
  [Ff] -> Subsystem6/2(F)
  Add Constant20/1 -> Subsystem12/1(index)
  [Ff] -> Subsystem2/2(F)
  [Ff] -> Subsystem18/2(F)
  Add Constant4/1 -> Add Constant5/1, Subsystem6/1(index)
  [Ff] -> Subsystem1/2(F)
  Add Constant5/1 -> Add Constant6/1, Subsystem7/1(index)
  Subsystem6/1(Out1) -> Add/6
  Add Constant12/1 -> Add Constant13/1, Subsystem18/1(index)
  Subsystem14/1(Out1) -> Add/13
  In1/1 -> Subsystem1/1(index), Add Constant/1
  [Ff] -> Subsystem16/2(F)
  Add Constant11/1 -> Add Constant14/1, Subsystem14/1(index)
  Add/1 -> Out1/1
  [Ff] -> Subsystem12/2(F)
  Subsystem17/1(Out1) -> Add/16
  Add Constant1/1 -> Add Constant2/1, Subsystem3/1(index)
  Subsystem10/1(Out1) -> Add/10
  Add Constant8/1 -> Add Constant9/1, Subsystem10/1(index)
  [Ff] -> Subsystem15/2(F)
  Add Constant10/1 -> Add Constant11/1, Subsystem13/1(index)
  Subsystem7/1(Out1) -> Add/7
  [Ff] -> Subsystem5/2(F)
  Add Constant7/1 -> Subsystem9/1(index), Add Constant8/1
  [Ff] -> Subsystem9/2(F)
  Subsystem9/1(Out1) -> Add/9
  [Ff] -> Subsystem17/2(F)
  [Ff] -> Subsystem3/2(F)
  Subsystem18/1(Out1) -> Add/17
  Subsystem12/1(Out1) -> Add/20
  In2/1 -> [Ff]

== system test03/Subsystem3/Subsystem3/Subsystem4 ==
  In2 : Inport
  In1 : Inport  Port=2
  Add : Sum  Inputs=++++++++++++++++++++
  Add Constant{,1..16,19..20} (19 alike) : Bias  Bias=1  SaturateOnIntegerOverflow=off
  Subsystem1 : Subsystem
  Subsystem10 : Subsystem
  Subsystem11 : Subsystem
  Subsystem12 : Subsystem
  Subsystem13 : Subsystem
  Subsystem14 : Subsystem
  Subsystem15 : Subsystem
  Subsystem16 : Subsystem
  Subsystem17 : Subsystem
  Subsystem18 : Subsystem
  Subsystem19 : Subsystem
  Subsystem2 : Subsystem
  Subsystem20 : Subsystem
  Subsystem3 : Subsystem
  Subsystem4 : Subsystem
  Subsystem5 : Subsystem
  Subsystem6 : Subsystem
  Subsystem7 : Subsystem
  Subsystem8 : Subsystem
  Subsystem9 : Subsystem
  Out1 : Outport
  wires:
  In2/1 -> [Ff]
  Subsystem12/1(Out1) -> Add/20
  Subsystem18/1(Out1) -> Add/17
  [Ff] -> Subsystem3/2(F)
  [Ff] -> Subsystem17/2(F)
  Subsystem9/1(Out1) -> Add/9
  [Ff] -> Subsystem9/2(F)
  Add Constant7/1 -> Add Constant8/1, Subsystem9/1(index)
  [Ff] -> Subsystem5/2(F)
  Subsystem7/1(Out1) -> Add/7
  Add Constant10/1 -> Subsystem13/1(index), Add Constant11/1
  [Ff] -> Subsystem15/2(F)
  Add Constant8/1 -> Subsystem10/1(index), Add Constant9/1
  Subsystem10/1(Out1) -> Add/10
  Add Constant1/1 -> Subsystem3/1(index), Add Constant2/1
  Subsystem17/1(Out1) -> Add/16
  [Ff] -> Subsystem12/2(F)
  Add/1 -> Out1/1
  Add Constant11/1 -> Subsystem14/1(index), Add Constant14/1
  [Ff] -> Subsystem16/2(F)
  In1/1 -> Add Constant/1, Subsystem1/1(index)
  Subsystem14/1(Out1) -> Add/13
  Add Constant12/1 -> Subsystem18/1(index), Add Constant13/1
  Subsystem6/1(Out1) -> Add/6
  Add Constant5/1 -> Subsystem7/1(index), Add Constant6/1
  [Ff] -> Subsystem1/2(F)
  Add Constant4/1 -> Subsystem6/1(index), Add Constant5/1
  [Ff] -> Subsystem18/2(F)
  [Ff] -> Subsystem2/2(F)
  Add Constant20/1 -> Subsystem12/1(index)
  [Ff] -> Subsystem6/2(F)
  Add Constant15/1 -> Subsystem16/1(index), Add Constant16/1
  [Ff] -> Subsystem19/2(F)
  Subsystem16/1(Out1) -> Add/15
  Subsystem20/1(Out1) -> Add/19
  [Ff] -> Subsystem10/2(F)
  [Ff] -> Subsystem13/2(F)
  Add Constant14/1 -> Subsystem15/1(index), Add Constant15/1
  [Ff] -> Subsystem7/2(F)
  Add Constant6/1 -> Subsystem8/1(index), Add Constant7/1
  Add Constant/1 -> Subsystem2/1(index), Add Constant1/1
  Subsystem2/1(Out1) -> Add/2
  [Ff] -> Subsystem11/2(F)
  Subsystem11/1(Out1) -> Add/11
  [Ff] -> Subsystem4/2(F)
  Subsystem15/1(Out1) -> Add/14
  Add Constant2/1 -> Subsystem4/1(index), Add Constant3/1
  Add Constant16/1 -> Subsystem17/1(index), Add Constant12/1
  Subsystem4/1(Out1) -> Add/4
  [Ff] -> Subsystem8/2(F)
  Subsystem3/1(Out1) -> Add/3
  Subsystem5/1(Out1) -> Add/5
  Subsystem8/1(Out1) -> Add/8
  [Ff] -> Subsystem14/2(F)
  Add Constant19/1 -> Add Constant20/1, Subsystem20/1(index)
  Add Constant9/1 -> Subsystem11/1(index), Add Constant10/1
  Add Constant3/1 -> Add Constant4/1, Subsystem5/1(index)
  Add Constant13/1 -> Subsystem19/1(index), Add Constant19/1
  Subsystem19/1(Out1) -> Add/18
  Subsystem13/1(Out1) -> Add/12
  [Ff] -> Subsystem20/2(F)
  Subsystem1/1(Out1) -> Add/1

== system test03/Subsystem8/Subsystem/Subsystem ==
  In1 : Inport
  In2 : Inport  Port=2
  Add : Sum  Inputs=++++++++++++++++++++
  Add Constant{,1..18} (19 alike) : Bias  Bias=1  SaturateOnIntegerOverflow=off
  Subsystem1 : Subsystem
  Subsystem10 : Subsystem
  Subsystem11 : Subsystem
  Subsystem12 : Subsystem
  Subsystem13 : Subsystem
  Subsystem14 : Subsystem
  Subsystem15 : Subsystem
  Subsystem16 : Subsystem
  Subsystem17 : Subsystem
  Subsystem18 : Subsystem
  Subsystem19 : Subsystem
  Subsystem2 : Subsystem
  Subsystem20 : Subsystem
  Subsystem3 : Subsystem
  Subsystem4 : Subsystem
  Subsystem5 : Subsystem
  Subsystem6 : Subsystem
  Subsystem7 : Subsystem
  Subsystem8 : Subsystem
  Subsystem9 : Subsystem
  Out1 : Outport
  wires:
  Subsystem14/1(Out1) -> Add/9
  Add Constant9/1 -> Add Constant10/1, Subsystem11/1(index)
  Add Constant2/1 -> Subsystem2/1(index), Add Constant3/1
  [Ff] -> Subsystem13/2(F)
  Add Constant11/1 -> Add Constant12/1, Subsystem16/1(index)
  Add Constant14/1 -> Add Constant15/1, Subsystem13/1(index)
  Subsystem8/1(Out1) -> Add/5
  [Ff] -> Subsystem17/2(F)
  Add Constant15/1 -> Add Constant16/1, Subsystem20/1(index)
  Subsystem1/1(Out1) -> Add/2
  Add Constant/1 -> Subsystem1/1(index), Add Constant1/1
  Add Constant3/1 -> Subsystem8/1(index), Add Constant4/1
  Subsystem9/1(Out1) -> Add/10
  [Ff] -> Subsystem3/2(F)
  Add Constant1/1 -> Subsystem3/1(index), Add Constant2/1
  Subsystem7/1(Out1) -> Add/7
  Add Constant17/1 -> Subsystem19/1(index), Add Constant18/1
  Add Constant18/1 -> Subsystem18/1(index)
  [Ff] -> Subsystem15/2(F)
  [Ff] -> Subsystem10/2(F)
  Subsystem5/1(Out1) -> Add/8
  Subsystem10/1(Out1) -> Add/12
  Subsystem6/1(Out1) -> Add/1
  Add Constant16/1 -> Subsystem17/1(index), Add Constant17/1
  Add Constant10/1 -> Add Constant11/1, Subsystem10/1(index)
  [Ff] -> Subsystem20/2(F)
  Subsystem17/1(Out1) -> Add/18
  Subsystem19/1(Out1) -> Add/19
  [Ff] -> Subsystem1/2(F)
  [Ff] -> Subsystem19/2(F)
  Add Constant8/1 -> Add Constant9/1, Subsystem9/1(index)
  [Ff] -> Subsystem2/2(F)
  Subsystem3/1(Out1) -> Add/3
  [Ff] -> Subsystem8/2(F)
  Subsystem20/1(Out1) -> Add/17
  Subsystem12/1(Out1) -> Add/14
  In1/1 -> Subsystem6/1(index), Add Constant/1
  Subsystem16/1(Out1) -> Add/13
  Subsystem13/1(Out1) -> Add/16
  [Ff] -> Subsystem6/2(F)
  [Ff] -> Subsystem7/2(F)
  [Ff] -> Subsystem11/2(F)
  Subsystem15/1(Out1) -> Add/15
  Add Constant13/1 -> Add Constant14/1, Subsystem15/1(index)
  Subsystem2/1(Out1) -> Add/4
  Add Constant12/1 -> Add Constant13/1, Subsystem12/1(index)
  Subsystem4/1(Out1) -> Add/6
  [Ff] -> Subsystem4/2(F)
  [Ff] -> Subsystem9/2(F)
  [Ff] -> Subsystem5/2(F)
  [Ff] -> Subsystem14/2(F)
  [Ff] -> Subsystem12/2(F)
  Subsystem18/1(Out1) -> Add/20
  Subsystem11/1(Out1) -> Add/11
  Add Constant5/1 -> Subsystem7/1(index), Add Constant6/1
  Add Constant6/1 -> Subsystem5/1(index), Add Constant7/1
  Add Constant4/1 -> Subsystem4/1(index), Add Constant5/1
  Add Constant7/1 -> Add Constant8/1, Subsystem14/1(index)
  [Ff] -> Subsystem16/2(F)
  [Ff] -> Subsystem18/2(F)
  Add/1 -> Out1/1
  In2/1 -> [Ff]

== system test03/Subsystem8/Subsystem/Subsystem1 ==
  In1 : Inport
  In2 : Inport  Port=2
  Add : Sum  Inputs=++++++++++++++++++++
  Add Constant{,1..18} (19 alike) : Bias  Bias=1  SaturateOnIntegerOverflow=off
  Subsystem1 : Subsystem
  Subsystem10 : Subsystem
  Subsystem11 : Subsystem
  Subsystem12 : Subsystem
  Subsystem13 : Subsystem
  Subsystem14 : Subsystem
  Subsystem15 : Subsystem
  Subsystem16 : Subsystem
  Subsystem17 : Subsystem
  Subsystem18 : Subsystem
  Subsystem19 : Subsystem
  Subsystem2 : Subsystem
  Subsystem20 : Subsystem
  Subsystem3 : Subsystem
  Subsystem4 : Subsystem
  Subsystem5 : Subsystem
  Subsystem6 : Subsystem
  Subsystem7 : Subsystem
  Subsystem8 : Subsystem
  Subsystem9 : Subsystem
  Out1 : Outport
  wires:
  In2/1 -> [Ff]
  Add/1 -> Out1/1
  [Ff] -> Subsystem18/2(F)
  [Ff] -> Subsystem16/2(F)
  Add Constant7/1 -> Subsystem14/1(index), Add Constant8/1
  Add Constant4/1 -> Add Constant5/1, Subsystem4/1(index)
  Add Constant6/1 -> Add Constant7/1, Subsystem5/1(index)
  Add Constant5/1 -> Add Constant6/1, Subsystem7/1(index)
  Subsystem11/1(Out1) -> Add/11
  Subsystem18/1(Out1) -> Add/20
  [Ff] -> Subsystem12/2(F)
  [Ff] -> Subsystem14/2(F)
  [Ff] -> Subsystem5/2(F)
  [Ff] -> Subsystem9/2(F)
  [Ff] -> Subsystem4/2(F)
  Subsystem4/1(Out1) -> Add/6
  Add Constant12/1 -> Subsystem12/1(index), Add Constant13/1
  Subsystem2/1(Out1) -> Add/4
  Add Constant13/1 -> Subsystem15/1(index), Add Constant14/1
  Subsystem15/1(Out1) -> Add/15
  [Ff] -> Subsystem11/2(F)
  [Ff] -> Subsystem7/2(F)
  [Ff] -> Subsystem6/2(F)
  Subsystem13/1(Out1) -> Add/16
  Subsystem16/1(Out1) -> Add/13
  In1/1 -> Add Constant/1, Subsystem6/1(index)
  Subsystem12/1(Out1) -> Add/14
  Subsystem20/1(Out1) -> Add/17
  [Ff] -> Subsystem8/2(F)
  Subsystem3/1(Out1) -> Add/3
  [Ff] -> Subsystem2/2(F)
  Add Constant8/1 -> Subsystem9/1(index), Add Constant9/1
  [Ff] -> Subsystem19/2(F)
  [Ff] -> Subsystem1/2(F)
  Subsystem19/1(Out1) -> Add/19
  Subsystem17/1(Out1) -> Add/18
  [Ff] -> Subsystem20/2(F)
  Add Constant10/1 -> Subsystem10/1(index), Add Constant11/1
  Add Constant16/1 -> Add Constant17/1, Subsystem17/1(index)
  Subsystem6/1(Out1) -> Add/1
  Subsystem10/1(Out1) -> Add/12
  Subsystem5/1(Out1) -> Add/8
  [Ff] -> Subsystem10/2(F)
  [Ff] -> Subsystem15/2(F)
  Add Constant18/1 -> Subsystem18/1(index)
  Add Constant17/1 -> Add Constant18/1, Subsystem19/1(index)
  Subsystem7/1(Out1) -> Add/7
  Add Constant1/1 -> Add Constant2/1, Subsystem3/1(index)
  [Ff] -> Subsystem3/2(F)
  Subsystem9/1(Out1) -> Add/10
  Add Constant3/1 -> Add Constant4/1, Subsystem8/1(index)
  Add Constant/1 -> Add Constant1/1, Subsystem1/1(index)
  Subsystem1/1(Out1) -> Add/2
  Add Constant15/1 -> Subsystem20/1(index), Add Constant16/1
  [Ff] -> Subsystem17/2(F)
  Subsystem8/1(Out1) -> Add/5
  Add Constant14/1 -> Subsystem13/1(index), Add Constant15/1
  Add Constant11/1 -> Subsystem16/1(index), Add Constant12/1
  [Ff] -> Subsystem13/2(F)
  Add Constant2/1 -> Add Constant3/1, Subsystem2/1(index)
  Add Constant9/1 -> Subsystem11/1(index), Add Constant10/1
  Subsystem14/1(Out1) -> Add/9

== system test03/Subsystem8/Subsystem/Subsystem2 ==
  In1 : Inport
  In2 : Inport  Port=2
  Add : Sum  Inputs=++++++++++++++++++++
  Add Constant{,1..18} (19 alike) : Bias  Bias=1  SaturateOnIntegerOverflow=off
  Subsystem1 : Subsystem
  Subsystem10 : Subsystem
  Subsystem11 : Subsystem
  Subsystem12 : Subsystem
  Subsystem13 : Subsystem
  Subsystem14 : Subsystem
  Subsystem15 : Subsystem
  Subsystem16 : Subsystem
  Subsystem17 : Subsystem
  Subsystem18 : Subsystem
  Subsystem19 : Subsystem
  Subsystem2 : Subsystem
  Subsystem20 : Subsystem
  Subsystem3 : Subsystem
  Subsystem4 : Subsystem
  Subsystem5 : Subsystem
  Subsystem6 : Subsystem
  Subsystem7 : Subsystem
  Subsystem8 : Subsystem
  Subsystem9 : Subsystem
  Out1 : Outport
  wires:
  Subsystem14/1(Out1) -> Add/9
  Add Constant9/1 -> Add Constant10/1, Subsystem11/1(index)
  Add Constant2/1 -> Subsystem2/1(index), Add Constant3/1
  [Ff] -> Subsystem13/2(F)
  Add Constant11/1 -> Add Constant12/1, Subsystem16/1(index)
  Add Constant14/1 -> Add Constant15/1, Subsystem13/1(index)
  Subsystem8/1(Out1) -> Add/5
  [Ff] -> Subsystem17/2(F)
  Add Constant15/1 -> Add Constant16/1, Subsystem20/1(index)
  Subsystem1/1(Out1) -> Add/2
  Add Constant/1 -> Subsystem1/1(index), Add Constant1/1
  Add Constant3/1 -> Subsystem8/1(index), Add Constant4/1
  Subsystem9/1(Out1) -> Add/10
  [Ff] -> Subsystem3/2(F)
  Add Constant1/1 -> Subsystem3/1(index), Add Constant2/1
  Subsystem7/1(Out1) -> Add/7
  Add Constant17/1 -> Subsystem19/1(index), Add Constant18/1
  Add Constant18/1 -> Subsystem18/1(index)
  [Ff] -> Subsystem15/2(F)
  [Ff] -> Subsystem10/2(F)
  Subsystem5/1(Out1) -> Add/8
  Subsystem10/1(Out1) -> Add/12
  Subsystem6/1(Out1) -> Add/1
  Add Constant16/1 -> Subsystem17/1(index), Add Constant17/1
  Add Constant10/1 -> Add Constant11/1, Subsystem10/1(index)
  [Ff] -> Subsystem20/2(F)
  Subsystem17/1(Out1) -> Add/18
  Subsystem19/1(Out1) -> Add/19
  [Ff] -> Subsystem1/2(F)
  [Ff] -> Subsystem19/2(F)
  Add Constant8/1 -> Add Constant9/1, Subsystem9/1(index)
  [Ff] -> Subsystem2/2(F)
  Subsystem3/1(Out1) -> Add/3
  [Ff] -> Subsystem8/2(F)
  Subsystem20/1(Out1) -> Add/17
  Subsystem12/1(Out1) -> Add/14
  In1/1 -> Subsystem6/1(index), Add Constant/1
  Subsystem16/1(Out1) -> Add/13
  Subsystem13/1(Out1) -> Add/16
  [Ff] -> Subsystem6/2(F)
  [Ff] -> Subsystem7/2(F)
  [Ff] -> Subsystem11/2(F)
  Subsystem15/1(Out1) -> Add/15
  Add Constant13/1 -> Add Constant14/1, Subsystem15/1(index)
  Subsystem2/1(Out1) -> Add/4
  Add Constant12/1 -> Add Constant13/1, Subsystem12/1(index)
  Subsystem4/1(Out1) -> Add/6
  [Ff] -> Subsystem4/2(F)
  [Ff] -> Subsystem9/2(F)
  [Ff] -> Subsystem5/2(F)
  [Ff] -> Subsystem14/2(F)
  [Ff] -> Subsystem12/2(F)
  Subsystem18/1(Out1) -> Add/20
  Subsystem11/1(Out1) -> Add/11
  Add Constant5/1 -> Subsystem7/1(index), Add Constant6/1
  Add Constant6/1 -> Subsystem5/1(index), Add Constant7/1
  Add Constant4/1 -> Subsystem4/1(index), Add Constant5/1
  Add Constant7/1 -> Add Constant8/1, Subsystem14/1(index)
  [Ff] -> Subsystem16/2(F)
  [Ff] -> Subsystem18/2(F)
  Add/1 -> Out1/1
  In2/1 -> [Ff]

== system test03/Subsystem8/Subsystem/Subsystem3 ==
  In1 : Inport
  In2 : Inport  Port=2
  Add : Sum  Inputs=++++++++++++++++++++
  Add Constant{,1..18} (19 alike) : Bias  Bias=1  SaturateOnIntegerOverflow=off
  Subsystem1 : Subsystem
  Subsystem10 : Subsystem
  Subsystem11 : Subsystem
  Subsystem12 : Subsystem
  Subsystem13 : Subsystem
  Subsystem14 : Subsystem
  Subsystem15 : Subsystem
  Subsystem16 : Subsystem
  Subsystem17 : Subsystem
  Subsystem18 : Subsystem
  Subsystem19 : Subsystem
  Subsystem2 : Subsystem
  Subsystem20 : Subsystem
  Subsystem3 : Subsystem
  Subsystem4 : Subsystem
  Subsystem5 : Subsystem
  Subsystem6 : Subsystem
  Subsystem7 : Subsystem
  Subsystem8 : Subsystem
  Subsystem9 : Subsystem
  Out1 : Outport
  wires:
  In2/1 -> [Ff]
  Add/1 -> Out1/1
  [Ff] -> Subsystem18/2(F)
  [Ff] -> Subsystem16/2(F)
  Add Constant7/1 -> Subsystem14/1(index), Add Constant8/1
  Add Constant4/1 -> Add Constant5/1, Subsystem4/1(index)
  Add Constant6/1 -> Add Constant7/1, Subsystem5/1(index)
  Add Constant5/1 -> Add Constant6/1, Subsystem7/1(index)
  Subsystem11/1(Out1) -> Add/11
  Subsystem18/1(Out1) -> Add/20
  [Ff] -> Subsystem12/2(F)
  [Ff] -> Subsystem14/2(F)
  [Ff] -> Subsystem5/2(F)
  [Ff] -> Subsystem9/2(F)
  [Ff] -> Subsystem4/2(F)
  Subsystem4/1(Out1) -> Add/6
  Add Constant12/1 -> Subsystem12/1(index), Add Constant13/1
  Subsystem2/1(Out1) -> Add/4
  Add Constant13/1 -> Subsystem15/1(index), Add Constant14/1
  Subsystem15/1(Out1) -> Add/15
  [Ff] -> Subsystem11/2(F)
  [Ff] -> Subsystem7/2(F)
  [Ff] -> Subsystem6/2(F)
  Subsystem13/1(Out1) -> Add/16
  Subsystem16/1(Out1) -> Add/13
  In1/1 -> Add Constant/1, Subsystem6/1(index)
  Subsystem12/1(Out1) -> Add/14
  Subsystem20/1(Out1) -> Add/17
  [Ff] -> Subsystem8/2(F)
  Subsystem3/1(Out1) -> Add/3
  [Ff] -> Subsystem2/2(F)
  Add Constant8/1 -> Subsystem9/1(index), Add Constant9/1
  [Ff] -> Subsystem19/2(F)
  [Ff] -> Subsystem1/2(F)
  Subsystem19/1(Out1) -> Add/19
  Subsystem17/1(Out1) -> Add/18
  [Ff] -> Subsystem20/2(F)
  Add Constant10/1 -> Subsystem10/1(index), Add Constant11/1
  Add Constant16/1 -> Add Constant17/1, Subsystem17/1(index)
  Subsystem6/1(Out1) -> Add/1
  Subsystem10/1(Out1) -> Add/12
  Subsystem5/1(Out1) -> Add/8
  [Ff] -> Subsystem10/2(F)
  [Ff] -> Subsystem15/2(F)
  Add Constant18/1 -> Subsystem18/1(index)
  Add Constant17/1 -> Add Constant18/1, Subsystem19/1(index)
  Subsystem7/1(Out1) -> Add/7
  Add Constant1/1 -> Add Constant2/1, Subsystem3/1(index)
  [Ff] -> Subsystem3/2(F)
  Subsystem9/1(Out1) -> Add/10
  Add Constant3/1 -> Add Constant4/1, Subsystem8/1(index)
  Add Constant/1 -> Add Constant1/1, Subsystem1/1(index)
  Subsystem1/1(Out1) -> Add/2
  Add Constant15/1 -> Subsystem20/1(index), Add Constant16/1
  [Ff] -> Subsystem17/2(F)
  Subsystem8/1(Out1) -> Add/5
  Add Constant14/1 -> Subsystem13/1(index), Add Constant15/1
  Add Constant11/1 -> Subsystem16/1(index), Add Constant12/1
  [Ff] -> Subsystem13/2(F)
  Add Constant2/1 -> Add Constant3/1, Subsystem2/1(index)
  Add Constant9/1 -> Subsystem11/1(index), Add Constant10/1
  Subsystem14/1(Out1) -> Add/9

== system test03/Subsystem8/Subsystem/Subsystem4 ==
  In1 : Inport
  In2 : Inport  Port=2
  Add : Sum  Inputs=++++++++++++++++++++
  Add Constant{,1..18} (19 alike) : Bias  Bias=1  SaturateOnIntegerOverflow=off
  Subsystem1 : Subsystem
  Subsystem10 : Subsystem
  Subsystem11 : Subsystem
  Subsystem12 : Subsystem
  Subsystem13 : Subsystem
  Subsystem14 : Subsystem
  Subsystem15 : Subsystem
  Subsystem16 : Subsystem
  Subsystem17 : Subsystem
  Subsystem18 : Subsystem
  Subsystem19 : Subsystem
  Subsystem2 : Subsystem
  Subsystem20 : Subsystem
  Subsystem3 : Subsystem
  Subsystem4 : Subsystem
  Subsystem5 : Subsystem
  Subsystem6 : Subsystem
  Subsystem7 : Subsystem
  Subsystem8 : Subsystem
  Subsystem9 : Subsystem
  Out1 : Outport
  wires:
  Subsystem14/1(Out1) -> Add/9
  Add Constant9/1 -> Add Constant10/1, Subsystem11/1(index)
  Add Constant2/1 -> Subsystem2/1(index), Add Constant3/1
  [Ff] -> Subsystem13/2(F)
  Add Constant11/1 -> Add Constant12/1, Subsystem16/1(index)
  Add Constant14/1 -> Add Constant15/1, Subsystem13/1(index)
  Subsystem8/1(Out1) -> Add/5
  [Ff] -> Subsystem17/2(F)
  Add Constant15/1 -> Add Constant16/1, Subsystem20/1(index)
  Subsystem1/1(Out1) -> Add/2
  Add Constant/1 -> Subsystem1/1(index), Add Constant1/1
  Add Constant3/1 -> Subsystem8/1(index), Add Constant4/1
  Subsystem9/1(Out1) -> Add/10
  [Ff] -> Subsystem3/2(F)
  Add Constant1/1 -> Subsystem3/1(index), Add Constant2/1
  Subsystem7/1(Out1) -> Add/7
  Add Constant17/1 -> Subsystem19/1(index), Add Constant18/1
  Add Constant18/1 -> Subsystem18/1(index)
  [Ff] -> Subsystem15/2(F)
  [Ff] -> Subsystem10/2(F)
  Subsystem5/1(Out1) -> Add/8
  Subsystem10/1(Out1) -> Add/12
  Subsystem6/1(Out1) -> Add/1
  Add Constant16/1 -> Subsystem17/1(index), Add Constant17/1
  Add Constant10/1 -> Add Constant11/1, Subsystem10/1(index)
  [Ff] -> Subsystem20/2(F)
  Subsystem17/1(Out1) -> Add/18
  Subsystem19/1(Out1) -> Add/19
  [Ff] -> Subsystem1/2(F)
  [Ff] -> Subsystem19/2(F)
  Add Constant8/1 -> Add Constant9/1, Subsystem9/1(index)
  [Ff] -> Subsystem2/2(F)
  Subsystem3/1(Out1) -> Add/3
  [Ff] -> Subsystem8/2(F)
  Subsystem20/1(Out1) -> Add/17
  Subsystem12/1(Out1) -> Add/14
  In1/1 -> Subsystem6/1(index), Add Constant/1
  Subsystem16/1(Out1) -> Add/13
  Subsystem13/1(Out1) -> Add/16
  [Ff] -> Subsystem6/2(F)
  [Ff] -> Subsystem7/2(F)
  [Ff] -> Subsystem11/2(F)
  Subsystem15/1(Out1) -> Add/15
  Add Constant13/1 -> Add Constant14/1, Subsystem15/1(index)
  Subsystem2/1(Out1) -> Add/4
  Add Constant12/1 -> Add Constant13/1, Subsystem12/1(index)
  Subsystem4/1(Out1) -> Add/6
  [Ff] -> Subsystem4/2(F)
  [Ff] -> Subsystem9/2(F)
  [Ff] -> Subsystem5/2(F)
  [Ff] -> Subsystem14/2(F)
  [Ff] -> Subsystem12/2(F)
  Subsystem18/1(Out1) -> Add/20
  Subsystem11/1(Out1) -> Add/11
  Add Constant5/1 -> Subsystem7/1(index), Add Constant6/1
  Add Constant6/1 -> Subsystem5/1(index), Add Constant7/1
  Add Constant4/1 -> Subsystem4/1(index), Add Constant5/1
  Add Constant7/1 -> Add Constant8/1, Subsystem14/1(index)
  [Ff] -> Subsystem16/2(F)
  [Ff] -> Subsystem18/2(F)
  Add/1 -> Out1/1
  In2/1 -> [Ff]
